simulink model "AcasXuClosedLoop_all_comp"  (Simulink 10.0)
settings: Solver=VariableStepAuto  StartTime=0.0  StopTime=20  RelTol=1e-3
libraries: neural x14040, simulink x2
(part 39 of 534)

== system AcasXuClosedLoop_all_comp/networks/Networks_m_9/AcasXu_5_9/Layer 3 ==
  "a{2} " : Inport  PortDimensions=50
      mask Display: e = 0.06;c1 = [120 230 180]/255;c2 = ([120 230 180]+0)/(256+128);c3 = ([120 230 180]+128)/(256+128);patch([0 1 1 0],[0 0 1 1],c1);patch([0 1 1 1-e 1-e 0+e],[0 0 1 1-e 0+e 0+e],c2);patch([0 0 1 1-e 0+e 0+e],[0 1 1 1-e 1-e 0+e],c3);plot([0 1 1 0 0],[0 0 1 1 0]);
  Delays 1 : Subsystem
      mask Display: e = 0.06;c1 = [120 150 230]/255;c2 = ([120 150 230]+0)/383;c3 = ([120 150 230]+128)/383;patch([0 1 1 0],[0 0 1 1],c1);patch([0 1 1 1-e 1-e 0+e],[0 0 1 1-e 0+e 0+e],c2);patch([0 0 1 1-e 0+e 0+e],[0 1 1 1-e 1-e 0+e],c3);plot([0 1 1 0 0],[0 0 1 1 0]);disp('0');
  "LW{3,2}" : Subsystem
      mask Display: e = 0.06;c1 = [120 150 230]/255;c2 = ([120 150 230]+0)/383;c3 = ([120 150 230]+128)/383;patch([0 1 1 0],[0 0 1 1],c1);patch([0 1 1 1-e 1-e 0+e],[0 0 1 1-e 0+e 0+e],c2);patch([0 0 1 1-e 0+e 0+e],[0 1 1 1-e 1-e 0+e],c3);plot([0 1 1 0 0],[0 0 1 1 0]);disp('W');
  "b{3}" : Constant  Value=[-1.4087099999999999067057387946988455951213836669921875;-0.303570999999999979745979317158344201743602752685546875;-0.028765800000000001090949552917663822881877422332763671875;-1.07050000000000000710542735760100185871124267578125;0.453349999999999975219822090366506017744541168212890625;0.52329499999999995463184632171760313212871551513671875;-1.7511900000000000243716158365714363753795623779296875;-0.2320919999999999927542404520863783545792102813720703125;-1.910320000000000018047785488306544721126556396484375;-0.21257300000000001194422338812728412449359893798828125;-0.009773469999999999380246862301646615378558635711669921875;-0.0103094999999999992201793475032900460064411163330078125;0.161032000000000008466116696581593714654445648193359375;-1.1012399999999999966604491419275291264057159423828125;1.32842999999999999971578290569595992565155029296875;-1.9569700000000000983391146291978657245635986328125;0.5231369999999999631512537234812043607234954833984375;-2.820329999999999781579163027345202863216400146484375;-1.4632199999999999651123516741790808737277984619140625;0.311817999999999984073184577937354333698749542236328125;-2.671460000000000167830194186535663902759552001953125;-0.0198090999999999996250554801235921331681311130523681640625;-0.703950999999999993406163412146270275115966796875;-0.006985049999999999592592558883552555926144123077392578125;-2.280600000000000182609483090345747768878936767578125;0.53753499999999998504307541224989108741283416748046875;-0.8202000000000000401456645704456605017185211181640625;-2.810869999999999979678477757261134684085845947265625;-0.026534599999999998465316508600153611041605472564697265625;-1.4842400000000000037658764995285309851169586181640625;-0.0947355000000000002646771690706373192369937896728515625;-1.2796499999999999541699935434735380113124847412109375;0.2260890000000000121804788477675174362957477569580078125;-0.11084199999999999608490952596184797585010528564453125;0.9424369999999999691908669774420559406280517578125;-0.50616899999999998005506540721398778259754180908203125;0.52418200000000003679900828501558862626552581787109375;-2.030879999999999796500560478307306766510009765625;1.08631000000000010885514711844734847545623779296875;0.62751199999999995871036162498057819902896881103515625;-3.177540000000000031121771826292388141155242919921875;-0.83951900000000001522693082733894698321819305419921875;0.60826700000000000212452277992269955575466156005859375;-0.91411699999999995736033042703638784587383270263671875;-0.53332100000000004502709316511754877865314483642578125;-2.010990000000000055280224842135794460773468017578125;1.0457300000000000483879603052628226578235626220703125;-0.54999600000000004040856538267689757049083709716796875;0.60113300000000002842881485776160843670368194580078125;0.035982300000000001782840541864061378873884677886962890625]
      mask Display: e = 0.06;c1 = [120 150 230]/255;c2 = ([120 150 230]+0)/383;c3 = ([120 150 230]+128)/383;patch([0 1 1 0],[0 0 1 1],c1);patch([0 1 1 1-e 1-e 0+e],[0 0 1 1-e 0+e 0+e],c2);patch([0 0 1 1-e 0+e 0+e],[0 1 1 1-e 1-e 0+e],c3);plot([0 1 1 0 0],[0 0 1 1 0]);disp('b');
  netsum : Sum  Inputs=2  SaturateOnIntegerOverflow=on  InputSameDT=on  OutDataTypeStr=Inherit: Same as first input
      mask Display: e = 0.06;c1 = [120 150 230]/255;c2 = ([120 150 230]+0)/383;c3 = ([120 150 230]+128)/383;patch([0 1 1 0],[0 0 1 1],c1);patch([0 1 1 1-e 1-e 0+e],[0 0 1 1-e 0+e 0+e],c2);patch([0 0 1 1-e 0+e 0+e],[0 1 1 1-e 1-e 0+e],c3);plot([0 1 1 0 0],[0 0 1 1 0]);disp('+');
  poslin : neural/Transfer Functions/poslin
      settings: SourceProductName=Deep Learning Toolbox  SourceProductBaseCode=NN
  "a{3}" : Outport  InitialOutput=[0;0;0;0;0;0;0;0;0;0;0;0;0;0;0;0;0;0;0;0;0;0;0;0;0;0;0;0;0;0;0;0;0;0;0;0;0;0;0;0;0;0;0;0;0;0;0;0;0;0]
      mask Display: e = 0.06;c1 = [120 230 180]/255;c2 = ([120 230 180]+0)/(256+128);c3 = ([120 230 180]+128)/(256+128);patch([0 1 1 0],[0 0 1 1],c1);patch([0 1 1 1-e 1-e 0+e],[0 0 1 1-e 0+e 0+e],c2);patch([0 0 1 1-e 0+e 0+e],[0 1 1 1-e 1-e 0+e],c3);plot([0 1 1 0 0],[0 0 1 1 0]);
  wires:
  poslin/1 -> "a{3}"/1
  netsum/1 -> poslin/1
  "b{3}"/1 -> netsum/2
  "LW{3,2}"/1("lz{3,2}") -> netsum/1
  Delays 1/1("pd{3,2}") -> "LW{3,2}"/1("ad{3,2}")
  "a{2} "/1 -> Delays 1/1("p{3}")

== system AcasXuClosedLoop_all_comp/networks/Networks_m_9/AcasXu_5_9/Layer 4 ==
  "a{3} " : Inport  PortDimensions=50
      mask Display: e = 0.06;c1 = [120 230 180]/255;c2 = ([120 230 180]+0)/(256+128);c3 = ([120 230 180]+128)/(256+128);patch([0 1 1 0],[0 0 1 1],c1);patch([0 1 1 1-e 1-e 0+e],[0 0 1 1-e 0+e 0+e],c2);patch([0 0 1 1-e 0+e 0+e],[0 1 1 1-e 1-e 0+e],c3);plot([0 1 1 0 0],[0 0 1 1 0]);
  Delays 1 : Subsystem
      mask Display: e = 0.06;c1 = [120 150 230]/255;c2 = ([120 150 230]+0)/383;c3 = ([120 150 230]+128)/383;patch([0 1 1 0],[0 0 1 1],c1);patch([0 1 1 1-e 1-e 0+e],[0 0 1 1-e 0+e 0+e],c2);patch([0 0 1 1-e 0+e 0+e],[0 1 1 1-e 1-e 0+e],c3);plot([0 1 1 0 0],[0 0 1 1 0]);disp('0');
  "LW{4,3}" : Subsystem
      mask Display: e = 0.06;c1 = [120 150 230]/255;c2 = ([120 150 230]+0)/383;c3 = ([120 150 230]+128)/383;patch([0 1 1 0],[0 0 1 1],c1);patch([0 1 1 1-e 1-e 0+e],[0 0 1 1-e 0+e 0+e],c2);patch([0 0 1 1-e 0+e 0+e],[0 1 1 1-e 1-e 0+e],c3);plot([0 1 1 0 0],[0 0 1 1 0]);disp('W');
  "b{4}" : Constant  Value=[3.772149999999999891997504164464771747589111328125;0.98809899999999994957278204310568980872631072998046875;-1.8413399999999999767652525406447239220142364501953125;1.3750899999999999234745473586372099816799163818359375;-2.300289999999999945856643535080365836620330810546875;0.2614170000000000104733999251038767397403717041015625;0.34013700000000002265920784338959492743015289306640625;-4.0836600000000000676436684443615376949310302734375;1.2628999999999999115374293978675268590450286865234375;-0.03431120000000000003215205879314453341066837310791015625;1.311150000000000037658764995285309851169586181640625;-3.46032999999999990592414178536273539066314697265625;-0.0169114999999999994495514243908473872579634189605712890625;-0.961446000000000022822632672614417970180511474609375;1.5270099999999999784705551064689643681049346923828125;5.4439399999999995571897670743055641651153564453125;0.62833200000000000162714286489062942564487457275390625;-0.01186710000000000021780355297096321010030806064605712890625;-1.3026400000000000201083594220108352601528167724609375;-0.07339779999999999915427650876154075376689434051513671875;2.089240000000000208046913030557334423065185546875;0.52902000000000004575895218295045197010040283203125;-0.03498110000000000108233422224657260812819004058837890625;-0.61602400000000001600852783667505718767642974853515625;4.40782999999999969276132105733267962932586669921875;-4.01264000000000020662582755903713405132293701171875;1.379140000000000032542857297812588512897491455078125;1.78906000000000009464429240324534475803375244140625;-3.38468999999999997640998117276467382907867431640625;0.09757639999999999369517666991669102571904659271240234375;1.3936299999999999243982529151253402233123779296875;1.0513799999999999812416717759333550930023193359375;-0.429449000000000025156765559586347080767154693603515625;-0.7367209999999999592290578220854513347148895263671875;-0.94990399999999997060484702160465531051158905029296875;1.5168500000000000316191517413244582712650299072265625;-0.91367399999999998616573293475084938108921051025390625;0.68007099999999998107824694670853205025196075439453125;0.83974000000000004195754854663391597568988800048828125;1.5033499999999999641175918441149406135082244873046875;-0.77747800000000000242295072894194163382053375244140625;-1.0734300000000001062971932697109878063201904296875;3.67147999999999985476506481063552200794219970703125;-1.231970000000000009521272659185342490673065185546875;-3.310799999999999965183405947755090892314910888671875;0.86703900000000000414246414948138408362865447998046875;0.21409800000000001052313791660708375275135040283203125;-0.96444600000000002548716793171479366719722747802734375;-0.0273295999999999990326404741836086031980812549591064453125;-2.31379000000000001335820343228988349437713623046875]
      mask Display: e = 0.06;c1 = [120 150 230]/255;c2 = ([120 150 230]+0)/383;c3 = ([120 150 230]+128)/383;patch([0 1 1 0],[0 0 1 1],c1);patch([0 1 1 1-e 1-e 0+e],[0 0 1 1-e 0+e 0+e],c2);patch([0 0 1 1-e 0+e 0+e],[0 1 1 1-e 1-e 0+e],c3);plot([0 1 1 0 0],[0 0 1 1 0]);disp('b');
  netsum : Sum  Inputs=2  SaturateOnIntegerOverflow=on  InputSameDT=on  OutDataTypeStr=Inherit: Same as first input
      mask Display: e = 0.06;c1 = [120 150 230]/255;c2 = ([120 150 230]+0)/383;c3 = ([120 150 230]+128)/383;patch([0 1 1 0],[0 0 1 1],c1);patch([0 1 1 1-e 1-e 0+e],[0 0 1 1-e 0+e 0+e],c2);patch([0 0 1 1-e 0+e 0+e],[0 1 1 1-e 1-e 0+e],c3);plot([0 1 1 0 0],[0 0 1 1 0]);disp('+');
  poslin : neural/Transfer Functions/poslin
      settings: SourceProductName=Deep Learning Toolbox  SourceProductBaseCode=NN
  "a{4}" : Outport  InitialOutput=[0;0;0;0;0;0;0;0;0;0;0;0;0;0;0;0;0;0;0;0;0;0;0;0;0;0;0;0;0;0;0;0;0;0;0;0;0;0;0;0;0;0;0;0;0;0;0;0;0;0]
      mask Display: e = 0.06;c1 = [120 230 180]/255;c2 = ([120 230 180]+0)/(256+128);c3 = ([120 230 180]+128)/(256+128);patch([0 1 1 0],[0 0 1 1],c1);patch([0 1 1 1-e 1-e 0+e],[0 0 1 1-e 0+e 0+e],c2);patch([0 0 1 1-e 0+e 0+e],[0 1 1 1-e 1-e 0+e],c3);plot([0 1 1 0 0],[0 0 1 1 0]);
  wires:
  poslin/1 -> "a{4}"/1
  netsum/1 -> poslin/1
  "b{4}"/1 -> netsum/2
  "LW{4,3}"/1("lz{4,3}") -> netsum/1
  Delays 1/1("pd{4,3}") -> "LW{4,3}"/1("ad{4,3}")
  "a{3} "/1 -> Delays 1/1("p{4}")

== system AcasXuClosedLoop_all_comp/networks/Networks_m_9/AcasXu_5_9/Layer 5 ==
  "a{4} " : Inport  PortDimensions=50
      mask Display: e = 0.06;c1 = [120 230 180]/255;c2 = ([120 230 180]+0)/(256+128);c3 = ([120 230 180]+128)/(256+128);patch([0 1 1 0],[0 0 1 1],c1);patch([0 1 1 1-e 1-e 0+e],[0 0 1 1-e 0+e 0+e],c2);patch([0 0 1 1-e 0+e 0+e],[0 1 1 1-e 1-e 0+e],c3);plot([0 1 1 0 0],[0 0 1 1 0]);
  Delays 1 : Subsystem
      mask Display: e = 0.06;c1 = [120 150 230]/255;c2 = ([120 150 230]+0)/383;c3 = ([120 150 230]+128)/383;patch([0 1 1 0],[0 0 1 1],c1);patch([0 1 1 1-e 1-e 0+e],[0 0 1 1-e 0+e 0+e],c2);patch([0 0 1 1-e 0+e 0+e],[0 1 1 1-e 1-e 0+e],c3);plot([0 1 1 0 0],[0 0 1 1 0]);disp('0');
  "LW{5,4}" : Subsystem
      mask Display: e = 0.06;c1 = [120 150 230]/255;c2 = ([120 150 230]+0)/383;c3 = ([120 150 230]+128)/383;patch([0 1 1 0],[0 0 1 1],c1);patch([0 1 1 1-e 1-e 0+e],[0 0 1 1-e 0+e 0+e],c2);patch([0 0 1 1-e 0+e 0+e],[0 1 1 1-e 1-e 0+e],c3);plot([0 1 1 0 0],[0 0 1 1 0]);disp('W');
  "b{5}" : Constant  Value=[4.529849999999999710098563809879124164581298828125;-0.0011309300000000000428757029879989204346202313899993896484375;-6.16978000000000026403768060845322906970977783203125;-0.74148800000000003596056785681867040693759918212890625;-1.127709999999999990194510246510617434978485107421875;-3.689670000000000005258016244624741375446319580078125;-2.24331000000000013727685654885135591030120849609375;0.81415800000000004832401145904441364109516143798828125;-5.4280699999999999505462255910970270633697509765625;-1.8660900000000000265032440438517369329929351806640625;-0.609118000000000048288484322256408631801605224609375;4.0063399999999997902477844036184251308441162109375;0.376890000000000002788880237858393229544162750244140625;0.424088999999999993750776638989918865263462066650390625;1.33898999999999990251353665371425449848175048828125;-0.769789999999999974278352965484373271465301513671875;-3.5713699999999999334931999328546226024627685546875;2.651120000000000143103306982084177434444427490234375;-4.66031999999999957395857563824392855167388916015625;0.65615100000000003976907692049280740320682525634765625;-2.396970000000000045048409447190351784229278564453125;0.84670400000000001217159706357051618397235870361328125;1.7186999999999998944844037396251223981380462646484375;0.73746999999999995889510273627820424735546112060546875;-0.68085399999999995923616324944305233657360076904296875;0.99495400000000000506616970596951432526111602783203125;-0.74764900000000000801492205937393009662628173828125;-1.0667500000000000870414851306122727692127227783203125;1.5786000000000000031974423109204508364200592041015625;0.54688199999999997924504668844747357070446014404296875;1.870870000000000032969182939268648624420166015625;1.487270000000000091944230007356964051723480224609375;-2.902969999999999828332875040359795093536376953125;-2.7686299999999999243982529151253402233123779296875;-4.68142999999999975813125274726189672946929931640625;1.5863199999999999523225824304972775280475616455078125;0.2463950000000000029043434324194095097482204437255859375;1.12568000000000001392663762089796364307403564453125;-8.1801600000000007639755494892597198486328125;2.570489999999999941593387120519764721393585205078125;1.7718700000000000560618218514719046652317047119140625;1.512140000000000039648284655413590371608734130859375;-0.333554999999999990389909498844644986093044281005859375;-0.055526100000000001843947217139429994858801364898681640625;-0.11815599999999999714095366698529687710106372833251953125;-0.1443190000000000028368418725221999920904636383056640625;-1.2461999999999999744204615126363933086395263671875;-7.360730000000000217141860048286616802215576171875;4.38281000000000009464429240324534475803375244140625;-5.64562999999999970413000482949428260326385498046875]
      mask Display: e = 0.06;c1 = [120 150 230]/255;c2 = ([120 150 230]+0)/383;c3 = ([120 150 230]+128)/383;patch([0 1 1 0],[0 0 1 1],c1);patch([0 1 1 1-e 1-e 0+e],[0 0 1 1-e 0+e 0+e],c2);patch([0 0 1 1-e 0+e 0+e],[0 1 1 1-e 1-e 0+e],c3);plot([0 1 1 0 0],[0 0 1 1 0]);disp('b');
  netsum : Sum  Inputs=2  SaturateOnIntegerOverflow=on  InputSameDT=on  OutDataTypeStr=Inherit: Same as first input
      mask Display: e = 0.06;c1 = [120 150 230]/255;c2 = ([120 150 230]+0)/383;c3 = ([120 150 230]+128)/383;patch([0 1 1 0],[0 0 1 1],c1);patch([0 1 1 1-e 1-e 0+e],[0 0 1 1-e 0+e 0+e],c2);patch([0 0 1 1-e 0+e 0+e],[0 1 1 1-e 1-e 0+e],c3);plot([0 1 1 0 0],[0 0 1 1 0]);disp('+');
  poslin : neural/Transfer Functions/poslin
      settings: SourceProductName=Deep Learning Toolbox  SourceProductBaseCode=NN
  "a{5}" : Outport  InitialOutput=[0;0;0;0;0;0;0;0;0;0;0;0;0;0;0;0;0;0;0;0;0;0;0;0;0;0;0;0;0;0;0;0;0;0;0;0;0;0;0;0;0;0;0;0;0;0;0;0;0;0]
      mask Display: e = 0.06;c1 = [120 230 180]/255;c2 = ([120 230 180]+0)/(256+128);c3 = ([120 230 180]+128)/(256+128);patch([0 1 1 0],[0 0 1 1],c1);patch([0 1 1 1-e 1-e 0+e],[0 0 1 1-e 0+e 0+e],c2);patch([0 0 1 1-e 0+e 0+e],[0 1 1 1-e 1-e 0+e],c3);plot([0 1 1 0 0],[0 0 1 1 0]);
  wires:
  poslin/1 -> "a{5}"/1
  netsum/1 -> poslin/1
  "b{5}"/1 -> netsum/2
  "LW{5,4}"/1("lz{5,4}") -> netsum/1
  Delays 1/1("pd{5,4}") -> "LW{5,4}"/1("ad{5,4}")
  "a{4} "/1 -> Delays 1/1("p{5}")

== system AcasXuClosedLoop_all_comp/networks/Networks_m_9/AcasXu_5_9/Layer 6 ==
  "a{5} " : Inport  PortDimensions=50
      mask Display: e = 0.06;c1 = [120 230 180]/255;c2 = ([120 230 180]+0)/(256+128);c3 = ([120 230 180]+128)/(256+128);patch([0 1 1 0],[0 0 1 1],c1);patch([0 1 1 1-e 1-e 0+e],[0 0 1 1-e 0+e 0+e],c2);patch([0 0 1 1-e 0+e 0+e],[0 1 1 1-e 1-e 0+e],c3);plot([0 1 1 0 0],[0 0 1 1 0]);
  Delays 1 : Subsystem
      mask Display: e = 0.06;c1 = [120 150 230]/255;c2 = ([120 150 230]+0)/383;c3 = ([120 150 230]+128)/383;patch([0 1 1 0],[0 0 1 1],c1);patch([0 1 1 1-e 1-e 0+e],[0 0 1 1-e 0+e 0+e],c2);patch([0 0 1 1-e 0+e 0+e],[0 1 1 1-e 1-e 0+e],c3);plot([0 1 1 0 0],[0 0 1 1 0]);disp('0');
  "LW{6,5}" : Subsystem
      mask Display: e = 0.06;c1 = [120 150 230]/255;c2 = ([120 150 230]+0)/383;c3 = ([120 150 230]+128)/383;patch([0 1 1 0],[0 0 1 1],c1);patch([0 1 1 1-e 1-e 0+e],[0 0 1 1-e 0+e 0+e],c2);patch([0 0 1 1-e 0+e 0+e],[0 1 1 1-e 1-e 0+e],c3);plot([0 1 1 0 0],[0 0 1 1 0]);disp('W');
  "b{6}" : Constant  Value=[0.70391499999999995740296299118199385702610015869140625;-5.26071000000000044138914745417423546314239501953125;1.4379500000000000614619466432486660778522491455078125;-8.31475999999999970668795867823064327239990234375;-0.11155400000000000038102854205135372467339038848876953125;-5.7538000000000000255795384873636066913604736328125;-11.6273999999999997356781022972427308559417724609375;-0.41080499999999997573496557379257865250110626220703125;-2.0769999999999999573674358543939888477325439453125;-0.9697350000000000136424205265939235687255859375;-9.465070000000000760564944357611238956451416015625;-3.28451000000000004064304448547773063182830810546875;-0.531730000000000035953462429461069405078887939453125;-8.7787000000000006139089236967265605926513671875;0.95654600000000000736832816983223892748355865478515625;0.84055500000000005211830966800334863364696502685546875;0.0288927999999999997327027045912473113276064395904541015625;0.68900499999999997857713651683297939598560333251953125;-0.88463400000000003142730520266923122107982635498046875;0.66112899999999996669686197492410428822040557861328125;-0.243254999999999999005240169935859739780426025390625;-8.03555000000000063664629124104976654052734375;-9.4148499999999994969357430818490684032440185546875;-5.65887000000000028876456781290471553802490234375;-3.653869999999999951256768326857127249240875244140625;-0.36082599999999998008348711664439179003238677978515625;-2.336629999999999984794385454733856022357940673828125;-0.0022638100000000002144651123359153643832542002201080322265625;-10.299099999999999255351212923415005207061767578125;-1.790350000000000108002495835535228252410888671875;-6.48200000000000020605739337042905390262603759765625;1.665929999999999910897940935683436691761016845703125;-0.6473430000000000017479351299698464572429656982421875;-5.207390000000000185309545486234128475189208984375;-1.3486100000000000864730509420041926205158233642578125;-1.020119999999999915729631538852117955684661865234375;-7.44967999999999985760723575367592275142669677734375;-2.162819999999999964757080306299030780792236328125;-1.4046099999999999141664375201798975467681884765625;-0.1722579999999999944559903042318182997405529022216796875;-6.11768000000000000682121026329696178436279296875;-8.94048000000000087084117694757878780364990234375;-7.65397999999999978371079123462550342082977294921875;-0.2140160000000000117825749157418613322079181671142578125;1.161399999999999987920773492078296840190887451171875;-4.0401600000000001955413608811795711517333984375;0.1396050000000000068656191842819680459797382354736328125;-4.3804999999999996163069226895458996295928955078125;-4.7943400000000000460431692772544920444488525390625;0.09888249999999999817479334751624264754354953765869140625]
      mask Display: e = 0.06;c1 = [120 150 230]/255;c2 = ([120 150 230]+0)/383;c3 = ([120 150 230]+128)/383;patch([0 1 1 0],[0 0 1 1],c1);patch([0 1 1 1-e 1-e 0+e],[0 0 1 1-e 0+e 0+e],c2);patch([0 0 1 1-e 0+e 0+e],[0 1 1 1-e 1-e 0+e],c3);plot([0 1 1 0 0],[0 0 1 1 0]);disp('b');
  netsum : Sum  Inputs=2  SaturateOnIntegerOverflow=on  InputSameDT=on  OutDataTypeStr=Inherit: Same as first input
      mask Display: e = 0.06;c1 = [120 150 230]/255;c2 = ([120 150 230]+0)/383;c3 = ([120 150 230]+128)/383;patch([0 1 1 0],[0 0 1 1],c1);patch([0 1 1 1-e 1-e 0+e],[0 0 1 1-e 0+e 0+e],c2);patch([0 0 1 1-e 0+e 0+e],[0 1 1 1-e 1-e 0+e],c3);plot([0 1 1 0 0],[0 0 1 1 0]);disp('+');
  poslin : neural/Transfer Functions/poslin
      settings: SourceProductName=Deep Learning Toolbox  SourceProductBaseCode=NN
  "a{6}" : Outport  InitialOutput=[0;0;0;0;0;0;0;0;0;0;0;0;0;0;0;0;0;0;0;0;0;0;0;0;0;0;0;0;0;0;0;0;0;0;0;0;0;0;0;0;0;0;0;0;0;0;0;0;0;0]
      mask Display: e = 0.06;c1 = [120 230 180]/255;c2 = ([120 230 180]+0)/(256+128);c3 = ([120 230 180]+128)/(256+128);patch([0 1 1 0],[0 0 1 1],c1);patch([0 1 1 1-e 1-e 0+e],[0 0 1 1-e 0+e 0+e],c2);patch([0 0 1 1-e 0+e 0+e],[0 1 1 1-e 1-e 0+e],c3);plot([0 1 1 0 0],[0 0 1 1 0]);
  wires:
  poslin/1 -> "a{6}"/1
  netsum/1 -> poslin/1
  "b{6}"/1 -> netsum/2
  "LW{6,5}"/1("lz{6,5}") -> netsum/1
  Delays 1/1("pd{6,5}") -> "LW{6,5}"/1("ad{6,5}")
  "a{5} "/1 -> Delays 1/1("p{6}")

== system AcasXuClosedLoop_all_comp/networks/Networks_m_9/AcasXu_5_9/Layer 7 ==
  "a{6} " : Inport  PortDimensions=50
      mask Display: e = 0.06;c1 = [120 230 180]/255;c2 = ([120 230 180]+0)/(256+128);c3 = ([120 230 180]+128)/(256+128);patch([0 1 1 0],[0 0 1 1],c1);patch([0 1 1 1-e 1-e 0+e],[0 0 1 1-e 0+e 0+e],c2);patch([0 0 1 1-e 0+e 0+e],[0 1 1 1-e 1-e 0+e],c3);plot([0 1 1 0 0],[0 0 1 1 0]);
  Delays 1 : Subsystem
      mask Display: e = 0.06;c1 = [120 150 230]/255;c2 = ([120 150 230]+0)/383;c3 = ([120 150 230]+128)/383;patch([0 1 1 0],[0 0 1 1],c1);patch([0 1 1 1-e 1-e 0+e],[0 0 1 1-e 0+e 0+e],c2);patch([0 0 1 1-e 0+e 0+e],[0 1 1 1-e 1-e 0+e],c3);plot([0 1 1 0 0],[0 0 1 1 0]);disp('0');
  "LW{7,6}" : Subsystem
      mask Display: e = 0.06;c1 = [120 150 230]/255;c2 = ([120 150 230]+0)/383;c3 = ([120 150 230]+128)/383;patch([0 1 1 0],[0 0 1 1],c1);patch([0 1 1 1-e 1-e 0+e],[0 0 1 1-e 0+e 0+e],c2);patch([0 0 1 1-e 0+e 0+e],[0 1 1 1-e 1-e 0+e],c3);plot([0 1 1 0 0],[0 0 1 1 0]);disp('W');
  "b{7}" : Constant  Value=[-0.020551300000000001511057945435823057778179645538330078125;0.0180315999999999983238740952629086677916347980499267578125;-0.0188092000000000016124435120445923530496656894683837890625;0.018163800000000000778310749183219741098582744598388671875;-0.0184214000000000009682477042360915220342576503753662109375]
      mask Display: e = 0.06;c1 = [120 150 230]/255;c2 = ([120 150 230]+0)/383;c3 = ([120 150 230]+128)/383;patch([0 1 1 0],[0 0 1 1],c1);patch([0 1 1 1-e 1-e 0+e],[0 0 1 1-e 0+e 0+e],c2);patch([0 0 1 1-e 0+e 0+e],[0 1 1 1-e 1-e 0+e],c3);plot([0 1 1 0 0],[0 0 1 1 0]);disp('b');
  netsum : Sum  Inputs=2  SaturateOnIntegerOverflow=on  InputSameDT=on  OutDataTypeStr=Inherit: Same as first input
      mask Display: e = 0.06;c1 = [120 150 230]/255;c2 = ([120 150 230]+0)/383;c3 = ([120 150 230]+128)/383;patch([0 1 1 0],[0 0 1 1],c1);patch([0 1 1 1-e 1-e 0+e],[0 0 1 1-e 0+e 0+e],c2);patch([0 0 1 1-e 0+e 0+e],[0 1 1 1-e 1-e 0+e],c3);plot([0 1 1 0 0],[0 0 1 1 0]);disp('+');
  purelin : neural/Transfer Functions/purelin
      settings: SourceProductName=Deep Learning Toolbox
  "a{7}" : Outport  InitialOutput=[0;0;0;0;0]
      mask Display: e = 0.06;c1 = [120 230 180]/255;c2 = ([120 230 180]+0)/(256+128);c3 = ([120 230 180]+128)/(256+128);patch([0 1 1 0],[0 0 1 1],c1);patch([0 1 1 1-e 1-e 0+e],[0 0 1 1-e 0+e 0+e],c2);patch([0 0 1 1-e 0+e 0+e],[0 1 1 1-e 1-e 0+e],c3);plot([0 1 1 0 0],[0 0 1 1 0]);
  wires:
  purelin/1 -> "a{7}"/1
  netsum/1 -> purelin/1
  "b{7}"/1 -> netsum/2
  "LW{7,6}"/1("lz{7,6}") -> netsum/1
  Delays 1/1("pd{7,6}") -> "LW{7,6}"/1("ad{7,6}")
  "a{6} "/1 -> Delays 1/1("p{7}")

== system AcasXuClosedLoop_all_comp/networks/Networks_m_9/AcasXu_5_9/Process Input 1 ==
  x : Inport  PortDimensions=5
      mask Display: e = 0.06;c1 = [120 230 180]/255;c2 = ([120 230 180]+0)/(256+128);c3 = ([120 230 180]+128)/(256+128);patch([0 1 1 0],[0 0 1 1],c1);patch([0 1 1 1-e 1-e 0+e],[0 0 1 1-e 0+e 0+e],c2);patch([0 0 1 1-e 0+e 0+e],[0 1 1 1-e 1-e 0+e],c3);plot([0 1 1 0 0],[0 0 1 1 0]);
  p : Outport  PortDimensions=5
      mask Display: e = 0.06;c1 = [120 230 180]/255;c2 = ([120 230 180]+0)/(256+128);c3 = ([120 230 180]+128)/(256+128);patch([0 1 1 0],[0 0 1 1],c1);patch([0 1 1 1-e 1-e 0+e],[0 0 1 1-e 0+e 0+e],c2);patch([0 0 1 1-e 0+e 0+e],[0 1 1 1-e 1-e 0+e],c3);plot([0 1 1 0 0],[0 0 1 1 0]);
  wires:
  x/1 -> p/1

== system AcasXuClosedLoop_all_comp/networks/Networks_m_9/AcasXu_5_9/Process Output 1 ==
  a : Inport  PortDimensions=5
      mask Display: e = 0.06;c1 = [120 230 180]/255;c2 = ([120 230 180]+0)/(256+128);c3 = ([120 230 180]+128)/(256+128);patch([0 1 1 0],[0 0 1 1],c1);patch([0 1 1 1-e 1-e 0+e],[0 0 1 1-e 0+e 0+e],c2);patch([0 0 1 1-e 0+e 0+e],[0 1 1 1-e 1-e 0+e],c3);plot([0 1 1 0 0],[0 0 1 1 0]);
  y : Outport  PortDimensions=5
      mask Display: e = 0.06;c1 = [120 230 180]/255;c2 = ([120 230 180]+0)/(256+128);c3 = ([120 230 180]+128)/(256+128);patch([0 1 1 0],[0 0 1 1],c1);patch([0 1 1 1-e 1-e 0+e],[0 0 1 1-e 0+e 0+e],c2);patch([0 0 1 1-e 0+e 0+e],[0 1 1 1-e 1-e 0+e],c3);plot([0 1 1 0 0],[0 0 1 1 0]);
  wires:
  a/1 -> y/1

== system AcasXuClosedLoop_all_comp/networks/Networks_m_1/AcasXu_1_1/Layer 1/Delays 1 ==
  "p{1}" : Inport  PortDimensions=5
      mask Display: e = 0.06;c1 = [120 230 180]/255;c2 = ([120 230 180]+0)/(256+128);c3 = ([120 230 180]+128)/(256+128);patch([0 1 1 0],[0 0 1 1],c1);patch([0 1 1 1-e 1-e 0+e],[0 0 1 1-e 0+e 0+e],c2);patch([0 0 1 1-e 0+e 0+e],[0 1 1 1-e 1-e 0+e],c3);plot([0 1 1 0 0],[0 0 1 1 0]);
  mux : Mux  Inputs=1
      mask Display: e = 0.06;c1 = [120 150 230]/255;c2 = ([120 150 230]+0)/383;c3 = ([120 150 230]+128)/383;patch([0 1 1 0],[0 0 1 1],c1);patch([0 1 1 1-e 1-e 0+e],[0 0 1 1-e 0+e 0+e],c2);patch([0 0 1 1-e 0+e 0+e],[0 1 1 1-e 1-e 0+e],c3);plot([0 1 1 0 0],[0 0 1 1 0]);disp('Mux')
  "pd{1,1}" : Outport
      mask Display: e = 0.06;c1 = [120 230 180]/255;c2 = ([120 230 180]+0)/(256+128);c3 = ([120 230 180]+128)/(256+128);patch([0 1 1 0],[0 0 1 1],c1);patch([0 1 1 1-e 1-e 0+e],[0 0 1 1-e 0+e 0+e],c2);patch([0 0 1 1-e 0+e 0+e],[0 1 1 1-e 1-e 0+e],c3);plot([0 1 1 0 0],[0 0 1 1 0]);
  wires:
  mux/1 -> "pd{1,1}"/1
  "p{1}"/1 -> mux/1
